simulink model "HvacHeatrTCtrl"  (R2022b)
settings: Solver=FixedStepDiscrete  StartTime=0.0  StopTime=10.0  FixedStep=0.2  RelTol=1e-3
(part 1 of 2)
settings Model: Creator=blue.ge  LastModifiedBy=blue.ge
settings DataIOCC: SaveFormat=StructureWithTime
settings OptimizationCC: DefaultParameterBehavior=Inlined  EnhancedBackFolding=on  PassReuseOutputArgsAs=Individual arguments  ZeroExternalMemoryAtStartup=off  ZeroInternalMemoryAtStartup=off  OptimizeBlockOrder=speed  DifferentSizesBufferReuse=on  OptimizationCustomize=off  EfficientTunableParamExpr=on
settings DebuggingCC: ParameterTunabilityLossMsg=error
settings ModelReferenceCC: ModelReferenceNumInstancesAllowed=Single
settings SFSimCC: MATLABDynamicMemAlloc=off
settings RTWCC: Description=AUTOSAR  SystemTargetFile=autosar.tlc  GenCodeOnly=on  TemplateMakefile=ert_default_tmf
settings CodeAppCC: ForceParamTrailComments=on  IgnoreCustomStorageClasses=off  OperatorAnnotations=on  SimulinkDataObjDesc=on  SFDataObjDesc=on  InsertBlockDesc=on
settings STFCustomTargetCC: TargetLangStandard=C99 (ISO)  MultiwordLength=256  ParMdlRefBuildCompliant=on  CompOptLevelCompliant=on  IncludeMdlTerminateFcn=off  CombineOutputUpdateFcns=on  SuppressErrorStatus=on  ERTCustomFileBanners=on  SupportNonFinite=off  SupportComplex=off  SupportContinuousTime=off  SupportNonInlinedSFcns=off  RemoveResetFunc=on  MATLABClassNameForMDSCustomization=Simulink.SoftwareTarget.ERTCustomization  AutosarCompliant=on  UseToolchainInfoCompliant=on  LookupTableObjectStructAxisOrder=2,1,3,4,...  LUTObjectStructOrderEvenSpacing=Size,Table,Breakpoints  SystemTargetFile=autosar.tlc
settings ERTTargetCC: TargetLangStandard=C99 (ISO)  ConcurrentExecutionCompliant=off  IncludeMdlTerminateFcn=off  SuppressErrorStatus=on  SupportNonFinite=off  SupportComplex=off  ModelStepFunctionPrototypeControlCompliant=off  CPPClassGenCompliant=off  AutosarCompliant=on  LookupTableObjectStructAxisOrder=2,1,3,4,...  LUTObjectStructOrderEvenSpacing=Size,Table,Breakpoints  InlinedParameterPlacement=NonHierarchical  ERTCustomFileTemplate=example_file_process.tlc

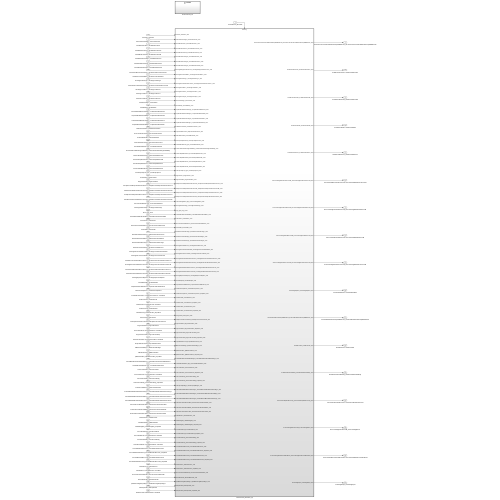
[thumbnail: Simulink's stored preview of the top-level diagram]
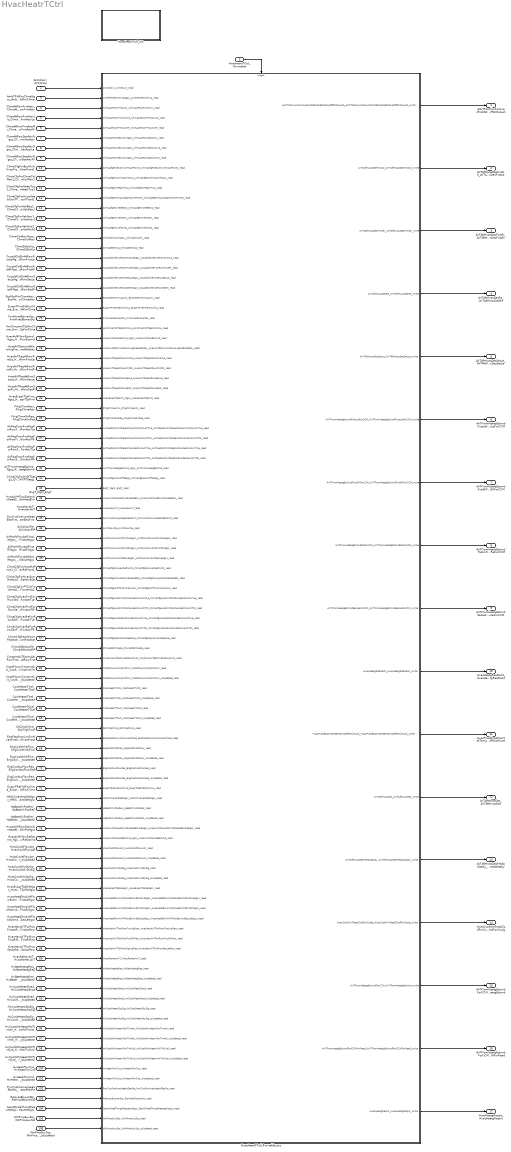
== system HvacHeatrTCtrl ==
  HvacHeatrTCtrl_Runnable : Inport  SampleTime=0.2  OutputFunctionCall=on
  AirCdnAvl_AirCdnAvl : Inport  Port=2  OutDataTypeStr=Boolean  PortDimensions=1
  AmbTFildForClimaNgcc_AmbTFildForClima : Inport  Port=3  OutDataTypeStr=T30  PortDimensions=1
  ClimaAtRowFrntActv_ClimaAtRowFrntActv : Inport  Port=4  OutDataTypeStr=Boolean  PortDimensions=1
  ClimaAtRowFrntActvLe_ClimaAtRowFrntActvLe : Inport  Port=5  OutDataTypeStr=Boolean  PortDimensions=1
  ClimaAtRowFrntActvRi_ClimaAtRowFrntActvRi : Inport  Port=6  OutDataTypeStr=Boolean  PortDimensions=1
  ClimaAtRowSecActvNgcc_ClimaAtRowSecActv : Inport  Port=7  OutDataTypeStr=Boolean  PortDimensions=1
  ClimaAtRowSecActvNgcc_ClimaAtRowSecActvLe : Inport  Port=8  OutDataTypeStr=Boolean  PortDimensions=1
  ClimaAtRowSecActvNgcc_ClimaAtRowSecActvRi : Inport  Port=9  OutDataTypeStr=Boolean  PortDimensions=1
  ClimaCfgForBypVlvInHvacPrsnt1_ClimaCfgForBypVlvInHvacPrsnt1 : Inport  Port=10  OutDataTypeStr=Boolean  PortDimensions=1
  ClimaCfgForClimaCtrlMan1_ClimaCfgForClimaCtrlMan1 : Inport  Port=11  OutDataTypeStr=Boolean  PortDimensions=1
  ClimaCfgForHeatrTyp1_ClimaCfgForHeatrTyp1 : Inport  Port=12  OutDataTypeStr=Enum: ClimaCfgForHeatrTyp1  PortDimensions=1
  ClimaCfgForHvacHeatrSnsrTPrsnt1_ClimaCfgForHvacHeatrSnsrTPrsnt1 : Inport  Port=13  OutDataTypeStr=Boolean  PortDimensions=1
  ClimaCfgForVehElec1_ClimaCfgForVehElec1 : Inport  Port=14  OutDataTypeStr=Boolean  PortDimensions=1
  ClimaCfgForVehHev1_ClimaCfgForVehHev1 : Inport  Port=15  OutDataTypeStr=Boolean  PortDimensions=1
  ClimaCfgForVehHyb1_ClimaCfgForVehHyb1 : Inport  Port=16  OutDataTypeStr=Boolean  PortDimensions=1
  ClimaCtrlActvNgcc_ClimaCtrlActv : Inport  Port=17  OutDataTypeStr=Boolean  PortDimensions=1
  ClimaStStrtUp_ClimaStStrtUp : Inport  Port=18  OutDataTypeStr=Enum: ClimaStStrtUp  PortDimensions=1
  CoupldCtrlErrAtRowFirstLeNgcc_CoupldCtrlErrAtRowFirstLe : Inport  Port=19  OutDataTypeStr=T30  PortDimensions=1
  CoupldCtrlErrAtRowFirstRiNgcc_CoupldCtrlErrAtRowFirstRi : Inport  Port=20  OutDataTypeStr=T30  PortDimensions=1
  CoupldCtrlErrAtRowSecLeNgcc_CoupldCtrlErrAtRowSecLe : Inport  Port=21  OutDataTypeStr=T30  PortDimensions=1
  CoupldCtrlErrAtRowSecRiNgcc_CoupldCtrlErrAtRowSecRi : Inport  Port=22  OutDataTypeStr=T30  PortDimensions=1
  EcoModForClimaActv_EcoModForClimaActv : Inport  Port=23  OutDataTypeStr=Enum: OnOff1  PortDimensions=1
  EvaprTFrntFildForClima_EvaprTFrntFildForClima : Inport  Port=24  OutDataTypeStr=T30  PortDimensions=1
  FrntHvacBlowerSts_FrntHvacBlowerSts : Inport  Port=25  OutDataTypeStr=Enum: HvacFanSts  PortDimensions=1
  HmiCmptmtTSpForClima_HmiCmptmtTSpForClima : Inport  Port=26  OutDataTypeStr=Bus: HmiCmptmtTSp  PortDimensions=1  BusOutputAsStruct=on
  HvacAirMFlowEstimd_Ngcc_HvacAirMFlowEstimd : Inport  Port=27  OutMin=0  OutMax=1000  OutDataTypeStr=MFlow1  PortDimensions=1
  HvacAirTEstimnAtRowAvgPredAtStaby_HvacAirTEstimnAtRowAvgPredAtStaby : Inport  Port=28  OutDataTypeStr=T30  PortDimensions=1
  HvacAirTReqAtRowFirstLe_HvacAirTReqAtRowFirstLe : Inport  Port=29  OutDataTypeStr=T30  PortDimensions=1
  HvacAirTReqAtRowFirstRi_HvacAirTReqAtRowFirstRi : Inport  Port=30  OutDataTypeStr=T30  PortDimensions=1
  HvacAirTReqAtRowSecLe_HvacAirTReqAtRowSecLe : Inport  Port=31  OutDataTypeStr=T30  PortDimensions=1
  HvacAirTReqAtRowSecRi_HvacAirTReqAtRowSecRi : Inport  Port=32  OutDataTypeStr=T30  PortDimensions=1
  HvacEvaprTSpFrnt_Ngcc_HvacEvaprTSpFrnt : Inport  Port=33  OutDataTypeStr=HvacEvaprTSp  PortDimensions=1
  PrkgClimaActv_PrkgClimaActv : Inport  Port=34  OutDataTypeStr=Enum: PrkgClimaActv  PortDimensions=1
  PrkgClimaOnReq_PrkgClimaOnReq : Inport  Port=35  OutDataTypeStr=Boolean  PortDimensions=1
  AirFlapPosnFrntReqForRowFirstForActrTLe_AirFlapPosnFrntReqForRowFirstForActrTLe : Inport  Port=36  OutMin=0  OutMax=100  OutDataTypeStr=Perc9  PortDimensions=1
  AirFlapPosnFrntReqForRowFirstForActrTRi_AirFlapPosnFrntReqForRowFirstForActrTRi : Inport  Port=37  OutMin=0  OutMax=100  OutDataTypeStr=Perc9  PortDimensions=1
  AirFlapPosnFrntReqForRowSecForActrTLe_AirFlapPosnFrntReqForRowSecForActrTLe : Inport  Port=38  OutMin=0  OutMax=100  OutDataTypeStr=Perc9  PortDimensions=1
  AirFlapPosnFrntReqForRowSecForActrTRi_AirFlapPosnFrntReqForRowSecForActrTRi : Inport  Port=39  OutMin=0  OutMax=100  OutDataTypeStr=Perc9  PortDimensions=1
  AirTFromHeatgEstimd_Ngcc_AirTFromHeatgEstimd : Inport  Port=40  OutDataTypeStr=T30  PortDimensions=1
  ClimaCfgForNrOfTSetg1_ClimaCfgForNrOfTSetg1 : Inport  Port=41  OutDataTypeStr=Enum: ClimaCfgForNrOfTSetg1  PortDimensions=1
  EngT_Ngcc_EngT : Inport  Port=42  OutDataTypeStr=Bus: EngT  PortDimensions=1  BusOutputAsStruct=on
  HvacAirMFlowEstimdInHeatElm_HvacAirMFlowEstimdInHeatElm : Inport  Port=43  OutDataTypeStr=AirFlow30  PortDimensions=1
  HvacHexAirT_HvacHexAirT : Inport  Port=44  OutDataTypeStr=Bus: HvacAirTForHeatrFrnt  PortDimensions=1  BusOutputAsStruct=on
  PwrCnsForHvacHeatrElecFrnt_PwrCnsForHvacHeatrElecFrnt : Inport  Port=45  OutDataTypeStr=Pwr33  PortDimensions=1
  AirCdnAvlRe_AirCdnAvlRe : Inport  Port=46  OutDataTypeStr=Boolean  PortDimensions=1
  AirPtcHVPwrActlFirstLeNgcc_AirPtcHVPwrActlFirstLeNgcc : Inport  Port=47  OutMin=0  OutMax=20460  OutDataTypeStr=Pwr3  PortDimensions=1
  AirPtcHVPwrActlFirstRiNgcc_AirPtcHVPwrActlFirstRiNgcc : Inport  Port=48  OutMin=0  OutMax=20460  OutDataTypeStr=Pwr3  PortDimensions=1
  AirPtcHVPwrActlSecLeNgcc_AirPtcHVPwrActlSecLeNgcc : Inport  Port=49  OutMin=0  OutMax=20460  OutDataTypeStr=Pwr3  PortDimensions=1
  ClimaCfgForHvacRePrsnt1_ClimaCfgForHvacRePrsnt1 : Inport  Port=50  OutDataTypeStr=Enum: CarCfgForHvacRe  PortDimensions=1
  ClimaCfgForHvacSnsrReHexESts_ClimaCfgForHvacSnsrReHexESts : Inport  Port=51  OutDataTypeStr=Enum: ClimaCfgForHvacSnsrReHexESts  PortDimensions=1
  ClimaCfgForPTCInFrontHVAC_ClimaCfgForPTCInFrontHVAC : Inport  Port=52  OutDataTypeStr=Enum: FrontHeaterExchanger  PortDimensions=1
  ClimaCfgHvacFrntForRowSecForActrTLe_ClimaCfgHvacFrntForRowSecForActrTLe : Inport  Port=53  OutDataTypeStr=Boolean  PortDimensions=1
  ClimaCfgHvacFrntForRowSecForActrTRi_ClimaCfgHvacFrntForRowSecForActrTRi : Inport  Port=54  OutDataTypeStr=Boolean  PortDimensions=1
  ClimaCfgHvacReForRowSecForActrTLe_ClimaCfgHvacReForRowSecForActrTLe : Inport  Port=55  OutDataTypeStr=UInt8  PortDimensions=1
  ClimaCfgHvacReForRowSecForActrTRi_ClimaCfgHvacReForRowSecForActrTRi : Inport  Port=56  OutDataTypeStr=UInt8  PortDimensions=1
  ClimaCfgReqVersionReplace_ClimaCfgReqVersionReplace : Inport  Port=57  OutDataTypeStr=UInt8  PortDimensions=1
  ClimaStStrtUpRe_ClimaStStrtUpRe : Inport  Port=58  OutDataTypeStr=Enum: ClimaStStrtUp  PortDimensions=1
  CmptmtAirTEstimdAtRowFirst_CmptmtAirTEstimdAtRowFirst : Inport  Port=59  OutDataTypeStr=T30  PortDimensions=1
  CooltFlowInCmptmtCirc_CooltFlowInCmptmtCirc : Inport  Port=60  OutDataTypeStr=HvacCooltFlow  PortDimensions=1
  CooltFlowInCmptmtCirc_CooltFlowInCmptmtCirc_IsUpdated : Inport  Port=61  OutDataTypeStr=boolean  PortDimensions=1
  CooltHeatrTIntk_CooltHeatrTIntk : Inport  Port=62  OutDataTypeStr=T6  PortDimensions=1
  CooltHeatrTIntk_CooltHeatrTIntk_IsUpdated : Inport  Port=63  OutDataTypeStr=boolean  PortDimensions=1
  CooltHeatrTOutl_CooltHeatrTOutl : Inport  Port=64  OutDataTypeStr=T6  PortDimensions=1
  CooltHeatrTOutl_CooltHeatrTOutl_IsUpdated : Inport  Port=65  OutDataTypeStr=boolean  PortDimensions=1
  DsChrgClima_DsChrgClima : Inport  Port=66  OutDataTypeStr=Boolean  PortDimensions=1
  EnaFlapPosnLimForHvacProtd_EnaFlapPosnLimForHvacProtd : Inport  Port=67  OutDataTypeStr=Boolean  PortDimensions=1
  EngCooltInhbFlow_EngCooltInhbFlow : Inport  Port=68  OutDataTypeStr=Enum: CooltInhb  PortDimensions=1
  EngCooltInhbFlow_EngCooltInhbFlow_IsUpdated : Inport  Port=69  OutDataTypeStr=boolean  PortDimensions=1
  EngCooltLoFlowReq_EngCooltLoFlowReq : Inport  Port=70  OutDataTypeStr=CooltLoFlow  PortDimensions=1
  EngCooltLoFlowReq_EngCooltLoFlowReq_IsUpdated : Inport  Port=71  OutDataTypeStr=boolean  PortDimensions=1
  EvaprTReFildForClima_EvaprTReFildForClima : Inport  Port=72  OutDataTypeStr=T30  PortDimensions=1
  HMIClimaVentSetNgcc_HMIClimaVentSetNgcc : Inport  Port=73  OutDataTypeStr=Enum: OnOff1  PortDimensions=1
  HpBattVlvPosRec_HpBattVlvPosRec : Inport  Port=74  OutMin=0  OutMax=100  OutDataTypeStr=Perc9  PortDimensions=1
  HpBattVlvPosRec_HpBattVlvPosRec_IsUpdated : Inport  Port=75  OutDataTypeStr=boolean  PortDimensions=1
  HvacAirMFlowEstimdInHeatElmReNgcc_HvacAirMFlowEstimdInHeatElmReNgcc : Inport  Port=76  OutDataTypeStr=AirFlow30  PortDimensions=1
  HvacAirMFlowReEstimd_Ngcc_HvacAirMFlowReEstimd : Inport  Port=77  OutMin=0  OutMax=1000  OutDataTypeStr=MFlow1  PortDimensions=1
  HvacCooltFlowAct_HvacCooltFlowAct : Inport  Port=78  OutDataTypeStr=HvacCooltFlow  PortDimensions=1
  HvacCooltFlowAct_HvacCooltFlowAct_IsUpdated : Inport  Port=79  OutDataTypeStr=boolean  PortDimensions=1
  HvacCooltVlvStsSig_HvacCooltVlvStsSig : Inport  Port=80  OutDataTypeStr=Enum: HvacCooltVlvSts  PortDimensions=1
  HvacCooltVlvStsSig_HvacCooltVlvStsSig_IsUpdated : Inport  Port=81  OutDataTypeStr=boolean  PortDimensions=1
  HvacEvaprTSpReNgcc_HvacEvaprTSpReNgcc : Inport  Port=82  OutDataTypeStr=HvacEvaprTSp  PortDimensions=1
  HvacHeatElmAirMFlowEstimdFirstLeNgcc_HvacHeatElmAirMFlowEstimdFirstLeNgcc : Inport  Port=83  OutDataTypeStr=AirFlow30  PortDimensions=1
  HvacHeatElmAirMFlowEstimdFirstRiNgcc_HvacHeatElmAirMFlowEstimdFirstRiNgcc : Inport  Port=84  OutDataTypeStr=AirFlow30  PortDimensions=1
  HvacHeatElmAirMFlowEstimdSecLeNgcc_HvacHeatElmAirMFlowEstimdSecLeNgcc : Inport  Port=85  OutDataTypeStr=AirFlow30  PortDimensions=1
  HvacHexAirTForRowFirstLeRaw_HvacHexAirTForRowFirstLeRaw : Inport  Port=86  OutDataTypeStr=Bus: HvacHexAirTForRowFirstLe  PortDimensions=1  BusOutputAsStruct=on
  HvacHexAirTForRowFirstRiRaw_HvacHexAirTForRowFirstRiRaw : Inport  Port=87  OutDataTypeStr=Bus: HvacHexAirTForRowFirstRi  PortDimensions=1  BusOutputAsStruct=on
  HvacHexAirTForRowSecLeRaw_HvacHexAirTForRowSecLeRaw : Inport  Port=88  OutDataTypeStr=Bus: HvacHexAirTForRowSecLe  PortDimensions=1  BusOutputAsStruct=on
  HvacReHexAirT_HvacReHexAirT : Inport  Port=89  OutDataTypeStr=Bus: HvacAirTForHeatrFrnt  PortDimensions=1  BusOutputAsStruct=on
  HvBattHeatgReq_HvBattHeatgReq : Inport  Port=90  OutDataTypeStr=Enum: HvBattCoolgReq  PortDimensions=1
  HvBattHeatgReq_HvBattHeatgReq_IsUpdated : Inport  Port=91  OutDataTypeStr=boolean  PortDimensions=1
  HvCooltHeatrEnad_HvCooltHeatrEnad : Inport  Port=92  OutDataTypeStr=Boolean  PortDimensions=1
  HvCooltHeatrEnad_HvCooltHeatrEnad_IsUpdated : Inport  Port=93  OutDataTypeStr=boolean  PortDimensions=1
  HvCooltHeatrStsSig_HvCooltHeatrStsSig : Inport  Port=94  OutDataTypeStr=Enum: HvCooltHeatrSts  PortDimensions=1
  HvCooltHeatrStsSig_HvCooltHeatrStsSig_IsUpdated : Inport  Port=95  OutDataTypeStr=boolean  PortDimensions=1
  HvCooltWtrHeatrWtrTInIntk_HvCooltWtrHeatrWtrTInIntk : Inport  Port=96  OutDataTypeStr=CooltT  PortDimensions=1
  HvCooltWtrHeatrWtrTInIntk_HvCooltWtrHeatrWtrTInIntk_IsUpdated : Inport  Port=97  OutDataTypeStr=boolean  PortDimensions=1
  HvCooltWtrHeatrWtrTInOutl_HvCooltWtrHeatrWtrTInOutl : Inport  Port=98  OutDataTypeStr=CooltT  PortDimensions=1
  HvCooltWtrHeatrWtrTInOutl_HvCooltWtrHeatrWtrTInOutl_IsUpdated : Inport  Port=99  OutDataTypeStr=boolean  PortDimensions=1
  HvHeatrPwrCns_HvHeatrPwrCns : Inport  Port=100  OutMin=0  OutMax=20460  OutDataTypeStr=Pwr3  PortDimensions=1
  HvHeatrPwrCns_HvHeatrPwrCns_IsUpdated : Inport  Port=101  OutDataTypeStr=boolean  PortDimensions=1
  PwrCnsForHvacHeatrElecRe_PwrCnsForHvacHeatrElecRe : Inport  Port=102  OutDataTypeStr=Pwr33  PortDimensions=1
  ReHvacBlowerSts_ReHvacBlowerSts : Inport  Port=103  OutDataTypeStr=Enum: HvacFanSts  PortDimensions=1
  SportModeThmgtRequestNgcc_SportModeThmgtRequestNgcc : Inport  Port=104  OutDataTypeStr=Enum: ThmgtLevel  PortDimensions=1
  WtrPmpAuxSts_WtrPmpAuxSts : Inport  Port=105  OutDataTypeStr=Enum: OnOffCrit1  PortDimensions=1
  WtrPmpAuxSts_WtrPmpAuxSts_IsUpdated : Inport  Port=106  OutDataTypeStr=boolean  PortDimensions=1
  HvacHeatrTCtrl_Init : Subsystem
  HvacHeatrTCtrl_Runnable_sys : Subsystem
  ActrTPosnLimForHvacProtdWhenSetTempDiffWithHvah_ActrTPosnLimForHvacProtdWhenSetTempDiffWithHvah : Outport  OutDataTypeStr=VCCPerc30  PortDimensions=1
  AirTAftHvacDesFirstLe_AirTAftHvacDesFirstLe : Outport  Port=2  OutDataTypeStr=T30  PortDimensions=1
  AirTAftHvacDesFirstRi_AirTAftHvacDesFirstRi : Outport  Port=3  OutDataTypeStr=T30  PortDimensions=1
  AirTAftHvacDesRe_AirTAftHvacDesRe : Outport  Port=4  OutDataTypeStr=T30  PortDimensions=1
  AirTAftHvacDesSecLe_AirTAftHvacDesSecLe : Outport  Port=5  OutDataTypeStr=T30  PortDimensions=1
  AirTFromHeatgEstimdFirstLeForCCM_AirTFromHeatgEstimdFirstLeForCCM : Outport  Port=6  OutDataTypeStr=T30  PortDimensions=1
  AirTFromHeatgEstimdFirstRiForCCM_AirTFromHeatgEstimdFirstRiForCCM : Outport  Port=7  OutDataTypeStr=T30  PortDimensions=1
  AirTFromHeatgEstimdReForCCM_AirTFromHeatgEstimdReForCCM : Outport  Port=8  OutDataTypeStr=T30  PortDimensions=1
  AirTFromHeatgEstimdSecLeForCCM_AirTFromHeatgEstimdSecLeForCCM : Outport  Port=9  OutDataTypeStr=T30  PortDimensions=1
  HvacHeatgReqReInt_HvacHeatgReqReInt : Outport  Port=10  OutDataTypeStr=Enum: OnOff1  PortDimensions=1
  HvacProtdEnaWhenSetTempDiffWithHvah_HvacProtdEnaWhenSetTempDiffWithHvah : Outport  Port=11  OutDataTypeStr=Boolean  PortDimensions=1
  AirTAftHvacDes_AirTAftHvacDes : Outport  Port=12  OutDataTypeStr=T30  PortDimensions=1
  AirTAftHvacDesPredAtStaby_AirTAftHvacDesPredAtStaby : Outport  Port=13  OutDataTypeStr=T30  PortDimensions=1
  HvacCooltVlvReqdClsdForCoolg_HvacCooltVlvReqdClsdForCoolg : Outport  Port=14  OutDataTypeStr=Boolean  PortDimensions=1
  AirTFromHeatgEstimdForCCM_AirTFromHeatgEstimd : Outport  Port=15  OutDataTypeStr=T30  PortDimensions=1
  AirTFromHeatgEstimdForCCMForRead_AirTFromHeatgEstimdForCCMForRead : Outport  Port=16  OutDataTypeStr=T30  PortDimensions=1
  HvacHeatgReqInt_HvacHeatgReqInt : Outport  Port=17  OutDataTypeStr=Enum: OnOff1  PortDimensions=1
  wires:
  AirCdnAvl_AirCdnAvl/1 -> HvacHeatrTCtrl_Runnable_sys/1(AirCdnAvl_AirCdnAvl_read)
  AmbTFildForClimaNgcc_AmbTFildForClima/1 -> HvacHeatrTCtrl_Runnable_sys/2(AmbTFildForClimaNgcc_AmbTFildForClima_read)
  ClimaAtRowFrntActv_ClimaAtRowFrntActv/1 -> HvacHeatrTCtrl_Runnable_sys/3(ClimaAtRowFrntActv_ClimaAtRowFrntActv_read)
  ClimaAtRowFrntActvLe_ClimaAtRowFrntActvLe/1 -> HvacHeatrTCtrl_Runnable_sys/4(ClimaAtRowFrntActvLe_ClimaAtRowFrntActvLe_read)
  ClimaAtRowFrntActvRi_ClimaAtRowFrntActvRi/1 -> HvacHeatrTCtrl_Runnable_sys/5(ClimaAtRowFrntActvRi_ClimaAtRowFrntActvRi_read)
  ClimaAtRowSecActvNgcc_ClimaAtRowSecActv/1 -> HvacHeatrTCtrl_Runnable_sys/6(ClimaAtRowSecActvNgcc_ClimaAtRowSecActv_read)
  ClimaAtRowSecActvNgcc_ClimaAtRowSecActvLe/1 -> HvacHeatrTCtrl_Runnable_sys/7(ClimaAtRowSecActvNgcc_ClimaAtRowSecActvLe_read)
  ClimaAtRowSecActvNgcc_ClimaAtRowSecActvRi/1 -> HvacHeatrTCtrl_Runnable_sys/8(ClimaAtRowSecActvNgcc_ClimaAtRowSecActvRi_read)
  ClimaCfgForBypVlvInHvacPrsnt1_ClimaCfgForBypVlvInHvacPrsnt1/1 -> HvacHeatrTCtrl_Runnable_sys/9(ClimaCfgForBypVlvInHvacPrsnt1_ClimaCfgForBypVlvInHvacPrsnt1_read)
  ClimaCfgForClimaCtrlMan1_ClimaCfgForClimaCtrlMan1/1 -> HvacHeatrTCtrl_Runnable_sys/10(ClimaCfgForClimaCtrlMan1_ClimaCfgForClimaCtrlMan1_read)
  ClimaCfgForHeatrTyp1_ClimaCfgForHeatrTyp1/1 -> HvacHeatrTCtrl_Runnable_sys/11(ClimaCfgForHeatrTyp1_ClimaCfgForHeatrTyp1_read)
  ClimaCfgForHvacHeatrSnsrTPrsnt1_ClimaCfgForHvacHeatrSnsrTPrsnt1/1 -> HvacHeatrTCtrl_Runnable_sys/12(ClimaCfgForHvacHeatrSnsrTPrsnt1_ClimaCfgForHvacHeatrSnsrTPrsnt1_read)
  ClimaCfgForVehElec1_ClimaCfgForVehElec1/1 -> HvacHeatrTCtrl_Runnable_sys/13(ClimaCfgForVehElec1_ClimaCfgForVehElec1_read)
  ClimaCfgForVehHev1_ClimaCfgForVehHev1/1 -> HvacHeatrTCtrl_Runnable_sys/14(ClimaCfgForVehHev1_ClimaCfgForVehHev1_read)
  ClimaCfgForVehHyb1_ClimaCfgForVehHyb1/1 -> HvacHeatrTCtrl_Runnable_sys/15(ClimaCfgForVehHyb1_ClimaCfgForVehHyb1_read)
  ClimaCtrlActvNgcc_ClimaCtrlActv/1 -> HvacHeatrTCtrl_Runnable_sys/16(ClimaCtrlActvNgcc_ClimaCtrlActv_read)
  ClimaStStrtUp_ClimaStStrtUp/1 -> HvacHeatrTCtrl_Runnable_sys/17(ClimaStStrtUp_ClimaStStrtUp_read)
  CoupldCtrlErrAtRowFirstLeNgcc_CoupldCtrlErrAtRowFirstLe/1 -> HvacHeatrTCtrl_Runnable_sys/18(CoupldCtrlErrAtRowFirstLeNgcc_CoupldCtrlErrAtRowFirstLe_read)
  CoupldCtrlErrAtRowFirstRiNgcc_CoupldCtrlErrAtRowFirstRi/1 -> HvacHeatrTCtrl_Runnable_sys/19(CoupldCtrlErrAtRowFirstRiNgcc_CoupldCtrlErrAtRowFirstRi_read)
  CoupldCtrlErrAtRowSecLeNgcc_CoupldCtrlErrAtRowSecLe/1 -> HvacHeatrTCtrl_Runnable_sys/20(CoupldCtrlErrAtRowSecLeNgcc_CoupldCtrlErrAtRowSecLe_read)
  CoupldCtrlErrAtRowSecRiNgcc_CoupldCtrlErrAtRowSecRi/1 -> HvacHeatrTCtrl_Runnable_sys/21(CoupldCtrlErrAtRowSecRiNgcc_CoupldCtrlErrAtRowSecRi_read)
  EcoModForClimaActv_EcoModForClimaActv/1 -> HvacHeatrTCtrl_Runnable_sys/22(EcoModForClimaActv_EcoModForClimaActv_read)
  EvaprTFrntFildForClima_EvaprTFrntFildForClima/1 -> HvacHeatrTCtrl_Runnable_sys/23(EvaprTFrntFildForClima_EvaprTFrntFildForClima_read)
  FrntHvacBlowerSts_FrntHvacBlowerSts/1 -> HvacHeatrTCtrl_Runnable_sys/24(FrntHvacBlowerSts_FrntHvacBlowerSts_read)
  HmiCmptmtTSpForClima_HmiCmptmtTSpForClima/1 -> HvacHeatrTCtrl_Runnable_sys/25(HmiCmptmtTSpForClima_HmiCmptmtTSpForClima_read)
  HvacAirMFlowEstimd_Ngcc_HvacAirMFlowEstimd/1 -> HvacHeatrTCtrl_Runnable_sys/26(HvacAirMFlowEstimd_Ngcc_HvacAirMFlowEstimd_read)
  HvacAirTEstimnAtRowAvgPredAtStaby_HvacAirTEstimnAtRowAvgPredAtStaby/1 -> HvacHeatrTCtrl_Runnable_sys/27(HvacAirTEstimnAtRowAvgPredAtStaby_HvacAirTEstimnAtRowAvgPredAtStaby_read)
  HvacAirTReqAtRowFirstLe_HvacAirTReqAtRowFirstLe/1 -> HvacHeatrTCtrl_Runnable_sys/28(HvacAirTReqAtRowFirstLe_HvacAirTReqAtRowFirstLe_read)
  HvacAirTReqAtRowFirstRi_HvacAirTReqAtRowFirstRi/1 -> HvacHeatrTCtrl_Runnable_sys/29(HvacAirTReqAtRowFirstRi_HvacAirTReqAtRowFirstRi_read)
  HvacAirTReqAtRowSecLe_HvacAirTReqAtRowSecLe/1 -> HvacHeatrTCtrl_Runnable_sys/30(HvacAirTReqAtRowSecLe_HvacAirTReqAtRowSecLe_read)
  HvacAirTReqAtRowSecRi_HvacAirTReqAtRowSecRi/1 -> HvacHeatrTCtrl_Runnable_sys/31(HvacAirTReqAtRowSecRi_HvacAirTReqAtRowSecRi_read)
  HvacEvaprTSpFrnt_Ngcc_HvacEvaprTSpFrnt/1 -> HvacHeatrTCtrl_Runnable_sys/32(HvacEvaprTSpFrnt_Ngcc_HvacEvaprTSpFrnt_read)
  PrkgClimaActv_PrkgClimaActv/1 -> HvacHeatrTCtrl_Runnable_sys/33(PrkgClimaActv_PrkgClimaActv_read)
  PrkgClimaOnReq_PrkgClimaOnReq/1 -> HvacHeatrTCtrl_Runnable_sys/34(PrkgClimaOnReq_PrkgClimaOnReq_read)
  AirFlapPosnFrntReqForRowFirstForActrTLe_AirFlapPosnFrntReqForRowFirstForActrTLe/1 -> HvacHeatrTCtrl_Runnable_sys/35(AirFlapPosnFrntReqForRowFirstForActrTLe_AirFlapPosnFrntReqForRowFirstForActrTLe_read)
  AirFlapPosnFrntReqForRowFirstForActrTRi_AirFlapPosnFrntReqForRowFirstForActrTRi/1 -> HvacHeatrTCtrl_Runnable_sys/36(AirFlapPosnFrntReqForRowFirstForActrTRi_AirFlapPosnFrntReqForRowFirstForActrTRi_read)
  AirFlapPosnFrntReqForRowSecForActrTLe_AirFlapPosnFrntReqForRowSecForActrTLe/1 -> HvacHeatrTCtrl_Runnable_sys/37(AirFlapPosnFrntReqForRowSecForActrTLe_AirFlapPosnFrntReqForRowSecForActrTLe_read)
  AirFlapPosnFrntReqForRowSecForActrTRi_AirFlapPosnFrntReqForRowSecForActrTRi/1 -> HvacHeatrTCtrl_Runnable_sys/38(AirFlapPosnFrntReqForRowSecForActrTRi_AirFlapPosnFrntReqForRowSecForActrTRi_read)
  AirTFromHeatgEstimd_Ngcc_AirTFromHeatgEstimd/1 -> HvacHeatrTCtrl_Runnable_sys/39(AirTFromHeatgEstimd_Ngcc_AirTFromHeatgEstimd_read)
  ClimaCfgForNrOfTSetg1_ClimaCfgForNrOfTSetg1/1 -> HvacHeatrTCtrl_Runnable_sys/40(ClimaCfgForNrOfTSetg1_ClimaCfgForNrOfTSetg1_read)
  EngT_Ngcc_EngT/1 -> HvacHeatrTCtrl_Runnable_sys/41(EngT_Ngcc_EngT_read)
  HvacAirMFlowEstimdInHeatElm_HvacAirMFlowEstimdInHeatElm/1 -> HvacHeatrTCtrl_Runnable_sys/42(HvacAirMFlowEstimdInHeatElm_HvacAirMFlowEstimdInHeatElm_read)
  HvacHexAirT_HvacHexAirT/1 -> HvacHeatrTCtrl_Runnable_sys/43(HvacHexAirT_HvacHexAirT_read)
  PwrCnsForHvacHeatrElecFrnt_PwrCnsForHvacHeatrElecFrnt/1 -> HvacHeatrTCtrl_Runnable_sys/44(PwrCnsForHvacHeatrElecFrnt_PwrCnsForHvacHeatrElecFrnt_read)
  AirCdnAvlRe_AirCdnAvlRe/1 -> HvacHeatrTCtrl_Runnable_sys/45(AirCdnAvlRe_AirCdnAvlRe_read)
  AirPtcHVPwrActlFirstLeNgcc_AirPtcHVPwrActlFirstLeNgcc/1 -> HvacHeatrTCtrl_Runnable_sys/46(AirPtcHVPwrActlFirstLeNgcc_AirPtcHVPwrActlFirstLeNgcc_read)
  AirPtcHVPwrActlFirstRiNgcc_AirPtcHVPwrActlFirstRiNgcc/1 -> HvacHeatrTCtrl_Runnable_sys/47(AirPtcHVPwrActlFirstRiNgcc_AirPtcHVPwrActlFirstRiNgcc_read)
  AirPtcHVPwrActlSecLeNgcc_AirPtcHVPwrActlSecLeNgcc/1 -> HvacHeatrTCtrl_Runnable_sys/48(AirPtcHVPwrActlSecLeNgcc_AirPtcHVPwrActlSecLeNgcc_read)
  ClimaCfgForHvacRePrsnt1_ClimaCfgForHvacRePrsnt1/1 -> HvacHeatrTCtrl_Runnable_sys/49(ClimaCfgForHvacRePrsnt1_ClimaCfgForHvacRePrsnt1_read)
  ClimaCfgForHvacSnsrReHexESts_ClimaCfgForHvacSnsrReHexESts/1 -> HvacHeatrTCtrl_Runnable_sys/50(ClimaCfgForHvacSnsrReHexESts_ClimaCfgForHvacSnsrReHexESts_read)
  ClimaCfgForPTCInFrontHVAC_ClimaCfgForPTCInFrontHVAC/1 -> HvacHeatrTCtrl_Runnable_sys/51(ClimaCfgForPTCInFrontHVAC_ClimaCfgForPTCInFrontHVAC_read)
  ClimaCfgHvacFrntForRowSecForActrTLe_ClimaCfgHvacFrntForRowSecForActrTLe/1 -> HvacHeatrTCtrl_Runnable_sys/52(ClimaCfgHvacFrntForRowSecForActrTLe_ClimaCfgHvacFrntForRowSecForActrTLe_read)
  ClimaCfgHvacFrntForRowSecForActrTRi_ClimaCfgHvacFrntForRowSecForActrTRi/1 -> HvacHeatrTCtrl_Runnable_sys/53(ClimaCfgHvacFrntForRowSecForActrTRi_ClimaCfgHvacFrntForRowSecForActrTRi_read)
  ClimaCfgHvacReForRowSecForActrTLe_ClimaCfgHvacReForRowSecForActrTLe/1 -> HvacHeatrTCtrl_Runnable_sys/54(ClimaCfgHvacReForRowSecForActrTLe_ClimaCfgHvacReForRowSecForActrTLe_read)
  ClimaCfgHvacReForRowSecForActrTRi_ClimaCfgHvacReForRowSecForActrTRi/1 -> HvacHeatrTCtrl_Runnable_sys/55(ClimaCfgHvacReForRowSecForActrTRi_ClimaCfgHvacReForRowSecForActrTRi_read)
  ClimaCfgReqVersionReplace_ClimaCfgReqVersionReplace/1 -> HvacHeatrTCtrl_Runnable_sys/56(ClimaCfgReqVersionReplace_ClimaCfgReqVersionReplace_read)
  ClimaStStrtUpRe_ClimaStStrtUpRe/1 -> HvacHeatrTCtrl_Runnable_sys/57(ClimaStStrtUpRe_ClimaStStrtUpRe_read)
  CmptmtAirTEstimdAtRowFirst_CmptmtAirTEstimdAtRowFirst/1 -> HvacHeatrTCtrl_Runnable_sys/58(CmptmtAirTEstimdAtRowFirst_CmptmtAirTEstimdAtRowFirst_read)
  CooltFlowInCmptmtCirc_CooltFlowInCmptmtCirc/1 -> HvacHeatrTCtrl_Runnable_sys/59(CooltFlowInCmptmtCirc_CooltFlowInCmptmtCirc_read)
  CooltFlowInCmptmtCirc_CooltFlowInCmptmtCirc_IsUpdated/1 -> HvacHeatrTCtrl_Runnable_sys/60(CooltFlowInCmptmtCirc_CooltFlowInCmptmtCirc_IsUpdated_read)
  CooltHeatrTIntk_CooltHeatrTIntk/1 -> HvacHeatrTCtrl_Runnable_sys/61(CooltHeatrTIntk_CooltHeatrTIntk_read)
  CooltHeatrTIntk_CooltHeatrTIntk_IsUpdated/1 -> HvacHeatrTCtrl_Runnable_sys/62(CooltHeatrTIntk_CooltHeatrTIntk_IsUpdated_read)
  CooltHeatrTOutl_CooltHeatrTOutl/1 -> HvacHeatrTCtrl_Runnable_sys/63(CooltHeatrTOutl_CooltHeatrTOutl_read)
  CooltHeatrTOutl_CooltHeatrTOutl_IsUpdated/1 -> HvacHeatrTCtrl_Runnable_sys/64(CooltHeatrTOutl_CooltHeatrTOutl_IsUpdated_read)
  DsChrgClima_DsChrgClima/1 -> HvacHeatrTCtrl_Runnable_sys/65(DsChrgClima_DsChrgClima_read)
  EnaFlapPosnLimForHvacProtd_EnaFlapPosnLimForHvacProtd/1 -> HvacHeatrTCtrl_Runnable_sys/66(EnaFlapPosnLimForHvacProtd_EnaFlapPosnLimForHvacProtd_read)
  EngCooltInhbFlow_EngCooltInhbFlow/1 -> HvacHeatrTCtrl_Runnable_sys/67(EngCooltInhbFlow_EngCooltInhbFlow_read)
  EngCooltInhbFlow_EngCooltInhbFlow_IsUpdated/1 -> HvacHeatrTCtrl_Runnable_sys/68(EngCooltInhbFlow_EngCooltInhbFlow_IsUpdated_read)
  EngCooltLoFlowReq_EngCooltLoFlowReq/1 -> HvacHeatrTCtrl_Runnable_sys/69(EngCooltLoFlowReq_EngCooltLoFlowReq_read)
  EngCooltLoFlowReq_EngCooltLoFlowReq_IsUpdated/1 -> HvacHeatrTCtrl_Runnable_sys/70(EngCooltLoFlowReq_EngCooltLoFlowReq_IsUpdated_read)
  EvaprTReFildForClima_EvaprTReFildForClima/1 -> HvacHeatrTCtrl_Runnable_sys/71(EvaprTReFildForClima_EvaprTReFildForClima_read)
  HMIClimaVentSetNgcc_HMIClimaVentSetNgcc/1 -> HvacHeatrTCtrl_Runnable_sys/72(HMIClimaVentSetNgcc_HMIClimaVentSetNgcc_read)
  HpBattVlvPosRec_HpBattVlvPosRec/1 -> HvacHeatrTCtrl_Runnable_sys/73(HpBattVlvPosRec_HpBattVlvPosRec_read)
  HpBattVlvPosRec_HpBattVlvPosRec_IsUpdated/1 -> HvacHeatrTCtrl_Runnable_sys/74(HpBattVlvPosRec_HpBattVlvPosRec_IsUpdated_read)
  HvacAirMFlowEstimdInHeatElmReNgcc_HvacAirMFlowEstimdInHeatElmReNgcc/1 -> HvacHeatrTCtrl_Runnable_sys/75(HvacAirMFlowEstimdInHeatElmReNgcc_HvacAirMFlowEstimdInHeatElmReNgcc_read)
  HvacAirMFlowReEstimd_Ngcc_HvacAirMFlowReEstimd/1 -> HvacHeatrTCtrl_Runnable_sys/76(HvacAirMFlowReEstimd_Ngcc_HvacAirMFlowReEstimd_read)
  HvacCooltFlowAct_HvacCooltFlowAct/1 -> HvacHeatrTCtrl_Runnable_sys/77(HvacCooltFlowAct_HvacCooltFlowAct_read)
  HvacCooltFlowAct_HvacCooltFlowAct_IsUpdated/1 -> HvacHeatrTCtrl_Runnable_sys/78(HvacCooltFlowAct_HvacCooltFlowAct_IsUpdated_read)
  HvacCooltVlvStsSig_HvacCooltVlvStsSig/1 -> HvacHeatrTCtrl_Runnable_sys/79(HvacCooltVlvStsSig_HvacCooltVlvStsSig_read)
  HvacCooltVlvStsSig_HvacCooltVlvStsSig_IsUpdated/1 -> HvacHeatrTCtrl_Runnable_sys/80(HvacCooltVlvStsSig_HvacCooltVlvStsSig_IsUpdated_read)
  HvacEvaprTSpReNgcc_HvacEvaprTSpReNgcc/1 -> HvacHeatrTCtrl_Runnable_sys/81(HvacEvaprTSpReNgcc_HvacEvaprTSpReNgcc_read)
  HvacHeatElmAirMFlowEstimdFirstLeNgcc_HvacHeatElmAirMFlowEstimdFirstLeNgcc/1 -> HvacHeatrTCtrl_Runnable_sys/82(HvacHeatElmAirMFlowEstimdFirstLeNgcc_HvacHeatElmAirMFlowEstimdFirstLeNgcc_read)
  HvacHeatElmAirMFlowEstimdFirstRiNgcc_HvacHeatElmAirMFlowEstimdFirstRiNgcc/1 -> HvacHeatrTCtrl_Runnable_sys/83(HvacHeatElmAirMFlowEstimdFirstRiNgcc_HvacHeatElmAirMFlowEstimdFirstRiNgcc_read)
  HvacHeatElmAirMFlowEstimdSecLeNgcc_HvacHeatElmAirMFlowEstimdSecLeNgcc/1 -> HvacHeatrTCtrl_Runnable_sys/84(HvacHeatElmAirMFlowEstimdSecLeNgcc_HvacHeatElmAirMFlowEstimdSecLeNgcc_read)
  HvacHexAirTForRowFirstLeRaw_HvacHexAirTForRowFirstLeRaw/1 -> HvacHeatrTCtrl_Runnable_sys/85(HvacHexAirTForRowFirstLeRaw_HvacHexAirTForRowFirstLeRaw_read)
  HvacHexAirTForRowFirstRiRaw_HvacHexAirTForRowFirstRiRaw/1 -> HvacHeatrTCtrl_Runnable_sys/86(HvacHexAirTForRowFirstRiRaw_HvacHexAirTForRowFirstRiRaw_read)
  HvacHexAirTForRowSecLeRaw_HvacHexAirTForRowSecLeRaw/1 -> HvacHeatrTCtrl_Runnable_sys/87(HvacHexAirTForRowSecLeRaw_HvacHexAirTForRowSecLeRaw_read)
  HvacReHexAirT_HvacReHexAirT/1 -> HvacHeatrTCtrl_Runnable_sys/88(HvacReHexAirT_HvacReHexAirT_read)
  HvBattHeatgReq_HvBattHeatgReq/1 -> HvacHeatrTCtrl_Runnable_sys/89(HvBattHeatgReq_HvBattHeatgReq_read)
  HvBattHeatgReq_HvBattHeatgReq_IsUpdated/1 -> HvacHeatrTCtrl_Runnable_sys/90(HvBattHeatgReq_HvBattHeatgReq_IsUpdated_read)
  HvCooltHeatrEnad_HvCooltHeatrEnad/1 -> HvacHeatrTCtrl_Runnable_sys/91(HvCooltHeatrEnad_HvCooltHeatrEnad_read)
  HvCooltHeatrEnad_HvCooltHeatrEnad_IsUpdated/1 -> HvacHeatrTCtrl_Runnable_sys/92(HvCooltHeatrEnad_HvCooltHeatrEnad_IsUpdated_read)
  HvCooltHeatrStsSig_HvCooltHeatrStsSig/1 -> HvacHeatrTCtrl_Runnable_sys/93(HvCooltHeatrStsSig_HvCooltHeatrStsSig_read)
  HvCooltHeatrStsSig_HvCooltHeatrStsSig_IsUpdated/1 -> HvacHeatrTCtrl_Runnable_sys/94(HvCooltHeatrStsSig_HvCooltHeatrStsSig_IsUpdated_read)
  HvCooltWtrHeatrWtrTInIntk_HvCooltWtrHeatrWtrTInIntk/1 -> HvacHeatrTCtrl_Runnable_sys/95(HvCooltWtrHeatrWtrTInIntk_HvCooltWtrHeatrWtrTInIntk_read)
  HvCooltWtrHeatrWtrTInIntk_HvCooltWtrHeatrWtrTInIntk_IsUpdated/1 -> HvacHeatrTCtrl_Runnable_sys/96(HvCooltWtrHeatrWtrTInIntk_HvCooltWtrHeatrWtrTInIntk_IsUpdated_read)
  HvCooltWtrHeatrWtrTInOutl_HvCooltWtrHeatrWtrTInOutl/1 -> HvacHeatrTCtrl_Runnable_sys/97(HvCooltWtrHeatrWtrTInOutl_HvCooltWtrHeatrWtrTInOutl_read)
  HvCooltWtrHeatrWtrTInOutl_HvCooltWtrHeatrWtrTInOutl_IsUpdated/1 -> HvacHeatrTCtrl_Runnable_sys/98(HvCooltWtrHeatrWtrTInOutl_HvCooltWtrHeatrWtrTInOutl_IsUpdated_read)
  HvHeatrPwrCns_HvHeatrPwrCns/1 -> HvacHeatrTCtrl_Runnable_sys/99(HvHeatrPwrCns_HvHeatrPwrCns_read)
  HvHeatrPwrCns_HvHeatrPwrCns_IsUpdated/1 -> HvacHeatrTCtrl_Runnable_sys/100(HvHeatrPwrCns_HvHeatrPwrCns_IsUpdated_read)
  PwrCnsForHvacHeatrElecRe_PwrCnsForHvacHeatrElecRe/1 -> HvacHeatrTCtrl_Runnable_sys/101(PwrCnsForHvacHeatrElecRe_PwrCnsForHvacHeatrElecRe_read)
  ReHvacBlowerSts_ReHvacBlowerSts/1 -> HvacHeatrTCtrl_Runnable_sys/102(ReHvacBlowerSts_ReHvacBlowerSts_read)
  SportModeThmgtRequestNgcc_SportModeThmgtRequestNgcc/1 -> HvacHeatrTCtrl_Runnable_sys/103(SportModeThmgtRequestNgcc_SportModeThmgtRequestNgcc_read)
  WtrPmpAuxSts_WtrPmpAuxSts/1 -> HvacHeatrTCtrl_Runnable_sys/104(WtrPmpAuxSts_WtrPmpAuxSts_read)
  WtrPmpAuxSts_WtrPmpAuxSts_IsUpdated/1 -> HvacHeatrTCtrl_Runnable_sys/105(WtrPmpAuxSts_WtrPmpAuxSts_IsUpdated_read)
  HvacHeatrTCtrl_Runnable_sys/1(ActrTPosnLimForHvacProtdWhenSetTempDiffWithHvah_ActrTPosnLimForHvacProtdWhenSetTempDiffWithHvah_write) -> ActrTPosnLimForHvacProtdWhenSetTempDiffWithHvah_ActrTPosnLimForHvacProtdWhenSetTempDiffWithHvah/1
  HvacHeatrTCtrl_Runnable_sys/2(AirTAftHvacDesFirstLe_AirTAftHvacDesFirstLe_write) -> AirTAftHvacDesFirstLe_AirTAftHvacDesFirstLe/1
  HvacHeatrTCtrl_Runnable_sys/3(AirTAftHvacDesFirstRi_AirTAftHvacDesFirstRi_write) -> AirTAftHvacDesFirstRi_AirTAftHvacDesFirstRi/1
  HvacHeatrTCtrl_Runnable_sys/4(AirTAftHvacDesRe_AirTAftHvacDesRe_write) -> AirTAftHvacDesRe_AirTAftHvacDesRe/1
  HvacHeatrTCtrl_Runnable_sys/5(AirTAftHvacDesSecLe_AirTAftHvacDesSecLe_write) -> AirTAftHvacDesSecLe_AirTAftHvacDesSecLe/1
  HvacHeatrTCtrl_Runnable_sys/6(AirTFromHeatgEstimdFirstLeForCCM_AirTFromHeatgEstimdFirstLeForCCM_write) -> AirTFromHeatgEstimdFirstLeForCCM_AirTFromHeatgEstimdFirstLeForCCM/1
  HvacHeatrTCtrl_Runnable_sys/7(AirTFromHeatgEstimdFirstRiForCCM_AirTFromHeatgEstimdFirstRiForCCM_write) -> AirTFromHeatgEstimdFirstRiForCCM_AirTFromHeatgEstimdFirstRiForCCM/1
  HvacHeatrTCtrl_Runnable_sys/8(AirTFromHeatgEstimdReForCCM_AirTFromHeatgEstimdReForCCM_write) -> AirTFromHeatgEstimdReForCCM_AirTFromHeatgEstimdReForCCM/1
  HvacHeatrTCtrl_Runnable_sys/9(AirTFromHeatgEstimdSecLeForCCM_AirTFromHeatgEstimdSecLeForCCM_write) -> AirTFromHeatgEstimdSecLeForCCM_AirTFromHeatgEstimdSecLeForCCM/1
  HvacHeatrTCtrl_Runnable_sys/10(HvacHeatgReqReInt_HvacHeatgReqReInt_write) -> HvacHeatgReqReInt_HvacHeatgReqReInt/1
  HvacHeatrTCtrl_Runnable_sys/11(HvacProtdEnaWhenSetTempDiffWithHvah_HvacProtdEnaWhenSetTempDiffWithHvah_write) -> HvacProtdEnaWhenSetTempDiffWithHvah_HvacProtdEnaWhenSetTempDiffWithHvah/1
  HvacHeatrTCtrl_Runnable_sys/12(AirTAftHvacDes_AirTAftHvacDes_write) -> AirTAftHvacDes_AirTAftHvacDes/1
  HvacHeatrTCtrl_Runnable_sys/13(AirTAftHvacDesPredAtStaby_AirTAftHvacDesPredAtStaby_write) -> AirTAftHvacDesPredAtStaby_AirTAftHvacDesPredAtStaby/1
  HvacHeatrTCtrl_Runnable_sys/14(HvacCooltVlvReqdClsdForCoolg_HvacCooltVlvReqdClsdForCoolg_write) -> HvacCooltVlvReqdClsdForCoolg_HvacCooltVlvReqdClsdForCoolg/1
  HvacHeatrTCtrl_Runnable_sys/15(AirTFromHeatgEstimdForCCM_AirTFromHeatgEstimd_write) -> AirTFromHeatgEstimdForCCM_AirTFromHeatgEstimd/1
  HvacHeatrTCtrl_Runnable_sys/16(AirTFromHeatgEstimdForCCMForRead_AirTFromHeatgEstimdForCCMForRead_write) -> AirTFromHeatgEstimdForCCMForRead_AirTFromHeatgEstimdForCCMForRead/1
  HvacHeatrTCtrl_Runnable_sys/17(HvacHeatgReqInt_HvacHeatgReqInt_write) -> HvacHeatgReqInt_HvacHeatgReqInt/1
  HvacHeatrTCtrl_Runnable/1 -> HvacHeatrTCtrl_Runnable_sys/Trigger

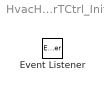
== system HvacHeatrTCtrl/HvacHeatrTCtrl_Init ==
  Event Listener : EventListener  EventName=reset
